simulink model "SdbRxSigProc"  (R2022b) (continued)
== system SdbRxSigProc == (continued)
  HvacFanSts3HvacFanBattI_HvacFanSts3HvacFanBattI/1 -> SdbRxSigProc_Runnable_sys/41(HvacFanSts3HvacFanBattI_HvacFanSts3HvacFanBattI_read)
  HvacFanSts3HvacFanSpdFd_HvacFanSts3HvacFanSpdFd/1 -> SdbRxSigProc_Runnable_sys/42(HvacFanSts3HvacFanSpdFd_HvacFanSts3HvacFanSpdFd_read)
  HvPwrEgyPrio_HvPwrEgyPrio/1 -> SdbRxSigProc_Runnable_sys/43(HvPwrEgyPrio_HvPwrEgyPrio_read)
  IHUAPSts_IHUAPSts/1 -> SdbRxSigProc_Runnable_sys/44(IHUAPSts_IHUAPSts_read)
  IncomingAirQlyCtrlFromHmi_IncomingAirQlyCtrlFromHmi/1 -> SdbRxSigProc_Runnable_sys/45(IncomingAirQlyCtrlFromHmi_IncomingAirQlyCtrlFromHmi_read)
  IncomingAirQlyPopUpReq_IncomingAirQlyPopUpReq/1 -> SdbRxSigProc_Runnable_sys/46(IncomingAirQlyPopUpReq_IncomingAirQlyPopUpReq_read)
  InPm25FanErr_InPm25FanErr/1 -> SdbRxSigProc_Runnable_sys/47(InPm25FanErr_InPm25FanErr_read)
  InPm25SnsrElecErr_InPm25SnsrElecErr/1 -> SdbRxSigProc_Runnable_sys/48(InPm25SnsrElecErr_InPm25SnsrElecErr_read)
  InPm25SnsrSts_InPm25SnsrSts/1 -> SdbRxSigProc_Runnable_sys/49(InPm25SnsrSts_InPm25SnsrSts_read)
  InteCleanUnpleSmell_InteCleanUnpleSmell/1 -> SdbRxSigProc_Runnable_sys/50(InteCleanUnpleSmell_InteCleanUnpleSmell_read)
  IsgMotT_IsgMotT/1 -> SdbRxSigProc_Runnable_sys/51(IsgMotT_IsgMotT_read)
  LeSolarData_LeSolarData/1 -> SdbRxSigProc_Runnable_sys/52(LeSolarData_LeSolarData_read)
  LockgCenSts_LockgCenSts/1 -> SdbRxSigProc_Runnable_sys/53(LockgCenSts_LockgCenSts_read)
  OnBdChrgrHndlSts1_OnBdChrgrHndlSts1/1 -> SdbRxSigProc_Runnable_sys/54(OnBdChrgrHndlSts1_OnBdChrgrHndlSts1_read)
  PassSeatHeatgActlPwrCns_PassSeatHeatgActlPwrCns/1 -> SdbRxSigProc_Runnable_sys/55(PassSeatHeatgActlPwrCns_PassSeatHeatgActlPwrCns_read)
  PassSeatHeatgDesPwrCns_PassSeatHeatgDesPwrCns/1 -> SdbRxSigProc_Runnable_sys/56(PassSeatHeatgDesPwrCns_PassSeatHeatgDesPwrCns_read)
  PassSeatVentnActlPwrCns_PassSeatVentnActlPwrCns/1 -> SdbRxSigProc_Runnable_sys/57(PassSeatVentnActlPwrCns_PassSeatVentnActlPwrCns_read)
  PassSeatVentnDesPwrCns_PassSeatVentnDesPwrCns/1 -> SdbRxSigProc_Runnable_sys/58(PassSeatVentnDesPwrCns_PassSeatVentnDesPwrCns_read)
  PostDrvgClimaReq_PostDrvgClimaReq/1 -> SdbRxSigProc_Runnable_sys/59(PostDrvgClimaReq_PostDrvgClimaReq_read)
  RainfallAmnt_RainfallAmnt/1 -> SdbRxSigProc_Runnable_sys/60(RainfallAmnt_RainfallAmnt_read)
  RelHumSnsrQf_RelHumSnsrQf/1 -> SdbRxSigProc_Runnable_sys/61(RelHumSnsrQf_RelHumSnsrQf_read)
  RemStrtHvCtrlReq_RemStrtHvCtrlReq/1 -> SdbRxSigProc_Runnable_sys/62(RemStrtHvCtrlReq_RemStrtHvCtrlReq_read)
  RiSolarData_RiSolarData/1 -> SdbRxSigProc_Runnable_sys/63(RiSolarData_RiSolarData_read)
  SceneModSeld_SceneModSeld/1 -> SdbRxSigProc_Runnable_sys/64(SceneModSeld_SceneModSeld_read)
  SeatHeatDurgClimaEnadFromTelm_SeatHeatDurgClimaEnadFromTelm/1 -> SdbRxSigProc_Runnable_sys/65(SeatHeatDurgClimaEnadFromTelm_SeatHeatDurgClimaEnadFromTelm_read)
  SecRowSeatHeatgActlPwrCnsLe_SecRowSeatHeatgActlPwrCnsLe/1 -> SdbRxSigProc_Runnable_sys/66(SecRowSeatHeatgActlPwrCnsLe_SecRowSeatHeatgActlPwrCnsLe_read)
  SecRowSeatHeatgActlPwrCnsRi_SecRowSeatHeatgActlPwrCnsRi/1 -> SdbRxSigProc_Runnable_sys/67(SecRowSeatHeatgActlPwrCnsRi_SecRowSeatHeatgActlPwrCnsRi_read)
  SecRowSeatHeatgDesPwrCnsLe_SecRowSeatHeatgDesPwrCnsLe/1 -> SdbRxSigProc_Runnable_sys/68(SecRowSeatHeatgDesPwrCnsLe_SecRowSeatHeatgDesPwrCnsLe_read)
  SecRowSeatHeatgDesPwrCnsRi_SecRowSeatHeatgDesPwrCnsRi/1 -> SdbRxSigProc_Runnable_sys/69(SecRowSeatHeatgDesPwrCnsRi_SecRowSeatHeatgDesPwrCnsRi_read)
  SecRowSeatVentnActlPwrCnsLe_SecRowSeatVentnActlPwrCnsLe/1 -> SdbRxSigProc_Runnable_sys/70(SecRowSeatVentnActlPwrCnsLe_SecRowSeatVentnActlPwrCnsLe_read)
  SecRowSeatVentnActlPwrCnsRi_SecRowSeatVentnActlPwrCnsRi/1 -> SdbRxSigProc_Runnable_sys/71(SecRowSeatVentnActlPwrCnsRi_SecRowSeatVentnActlPwrCnsRi_read)
  SecRowSeatVentnDesPwrCnsLe_SecRowSeatVentnDesPwrCnsLe/1 -> SdbRxSigProc_Runnable_sys/72(SecRowSeatVentnDesPwrCnsLe_SecRowSeatVentnDesPwrCnsLe_read)
  SecRowSeatVentnDesPwrCnsRi_SecRowSeatVentnDesPwrCnsRi/1 -> SdbRxSigProc_Runnable_sys/73(SecRowSeatVentnDesPwrCnsRi_SecRowSeatVentnDesPwrCnsRi_read)
  SolarSnsrVluQf_SolarSnsrVluQf/1 -> SdbRxSigProc_Runnable_sys/74(SolarSnsrVluQf_SolarSnsrVluQf_read)
  SteerWhlHeatgDesPwr_SteerWhlHeatgDesPwr/1 -> SdbRxSigProc_Runnable_sys/75(SteerWhlHeatgDesPwr_SteerWhlHeatgDesPwr_read)
  SteerWhlHeatgPwrAct_SteerWhlHeatgPwrAct/1 -> SdbRxSigProc_Runnable_sys/76(SteerWhlHeatgPwrAct_SteerWhlHeatgPwrAct_read)
  StrtRemSts1WdStrtRemSts_StrtRemSts1WdStrtRemSts/1 -> SdbRxSigProc_Runnable_sys/77(StrtRemSts1WdStrtRemSts_StrtRemSts1WdStrtRemSts_read)
  SunCurtPosnSts_SunCurtPosnSts/1 -> SdbRxSigProc_Runnable_sys/78(SunCurtPosnSts_SunCurtPosnSts_read)
  SunRoofPosnSts_SunRoofPosnSts/1 -> SdbRxSigProc_Runnable_sys/79(SunRoofPosnSts_SunRoofPosnSts_read)
  TelmPM25Req_TelmPM25Req/1 -> SdbRxSigProc_Runnable_sys/80(TelmPM25Req_TelmPM25Req_read)
  TrSts_TrSts/1 -> SdbRxSigProc_Runnable_sys/81(TrSts_TrSts_read)
  VehBattU_VehBattU/1 -> SdbRxSigProc_Runnable_sys/82(VehBattU_VehBattU_read)
  VehSpdLgt_VehSpdLgt/1 -> SdbRxSigProc_Runnable_sys/83(VehSpdLgt_VehSpdLgt_read)
  VehTiAndData_VehTiAndData/1 -> SdbRxSigProc_Runnable_sys/84(VehTiAndData_VehTiAndData_read)
  WadeProtnSts_WadeProtnSts/1 -> SdbRxSigProc_Runnable_sys/85(WadeProtnSts_WadeProtnSts_read)
  WinGlbCmd_WinGlbCmd/1 -> SdbRxSigProc_Runnable_sys/86(WinGlbCmd_WinGlbCmd_read)
  WinPosnStsAtDrvr_WinPosnStsAtDrvr/1 -> SdbRxSigProc_Runnable_sys/87(WinPosnStsAtDrvr_WinPosnStsAtDrvr_read)
  WinPosnStsAtPass_WinPosnStsAtPass/1 -> SdbRxSigProc_Runnable_sys/88(WinPosnStsAtPass_WinPosnStsAtPass_read)
  WinPosnStsAtReLe_WinPosnStsAtReLe/1 -> SdbRxSigProc_Runnable_sys/89(WinPosnStsAtReLe_WinPosnStsAtReLe_read)
  WinPosnStsAtReRi_WinPosnStsAtReRi/1 -> SdbRxSigProc_Runnable_sys/90(WinPosnStsAtReRi_WinPosnStsAtReRi_read)
  WipgInfo_WipgInfo/1 -> SdbRxSigProc_Runnable_sys/91(WipgInfo_WipgInfo_read)
  VehModMngtGlbSafe1_VehModMngtGlbSafe1/1 -> SdbRxSigProc_Runnable_sys/92(VehModMngtGlbSafe1_VehModMngtGlbSafe1_read)
  VehModMngtGlbSafe1_VehModMngtGlbSafe1_IsUpdated/1 -> SdbRxSigProc_Runnable_sys/93(VehModMngtGlbSafe1_VehModMngtGlbSafe1_IsUpdated_read)
  AACAirClngErrSts_AACAirClngErrSts/1 -> SdbRxSigProc_Runnable_sys/94(AACAirClngErrSts_AACAirClngErrSts_read)
  AACAirClngSts_AACAirClngSts/1 -> SdbRxSigProc_Runnable_sys/95(AACAirClngSts_AACAirClngSts_read)
  AFUFragraCh1AvlTiPrec_AFUFragraCh1AvlTiPrec/1 -> SdbRxSigProc_Runnable_sys/96(AFUFragraCh1AvlTiPrec_AFUFragraCh1AvlTiPrec_read)
  AFUFragraCh1AvlTiPrec_AFUFragraCh1AvlTiPrec_IsUpdated/1 -> SdbRxSigProc_Runnable_sys/97(AFUFragraCh1AvlTiPrec_AFUFragraCh1AvlTiPrec_IsUpdated_read)
  AFUFragraCh2AvlTiPrec_AFUFragraCh2AvlTiPrec/1 -> SdbRxSigProc_Runnable_sys/98(AFUFragraCh2AvlTiPrec_AFUFragraCh2AvlTiPrec_read)
  AFUFragraCh2AvlTiPrec_AFUFragraCh2AvlTiPrec_IsUpdated/1 -> SdbRxSigProc_Runnable_sys/99(AFUFragraCh2AvlTiPrec_AFUFragraCh2AvlTiPrec_IsUpdated_read)
  AFUFragraCh3AvlTiPrec_AFUFragraCh3AvlTiPrec/1 -> SdbRxSigProc_Runnable_sys/100(AFUFragraCh3AvlTiPrec_AFUFragraCh3AvlTiPrec_read)
  AFUFragraCh3AvlTiPrec_AFUFragraCh3AvlTiPrec_IsUpdated/1 -> SdbRxSigProc_Runnable_sys/101(AFUFragraCh3AvlTiPrec_AFUFragraCh3AvlTiPrec_IsUpdated_read)
  AFUFragraCh4AvlTiPrec_AFUFragraCh4AvlTiPrec/1 -> SdbRxSigProc_Runnable_sys/102(AFUFragraCh4AvlTiPrec_AFUFragraCh4AvlTiPrec_read)
  AFUFragraCh4AvlTiPrec_AFUFragraCh4AvlTiPrec_IsUpdated/1 -> SdbRxSigProc_Runnable_sys/103(AFUFragraCh4AvlTiPrec_AFUFragraCh4AvlTiPrec_IsUpdated_read)
  AFUFragraCh5AvlTiPrec_AFUFragraCh5AvlTiPrec/1 -> SdbRxSigProc_Runnable_sys/104(AFUFragraCh5AvlTiPrec_AFUFragraCh5AvlTiPrec_read)
  AFUFragraCh5AvlTiPrec_AFUFragraCh5AvlTiPrec_IsUpdated/1 -> SdbRxSigProc_Runnable_sys/105(AFUFragraCh5AvlTiPrec_AFUFragraCh5AvlTiPrec_IsUpdated_read)
  AirFragCh1OvrdDy_AirFragCh1OvrdDy/1 -> SdbRxSigProc_Runnable_sys/106(AirFragCh1OvrdDy_AirFragCh1OvrdDy_read)
  AirFragCh2OvrdDy_AirFragCh2OvrdDy/1 -> SdbRxSigProc_Runnable_sys/107(AirFragCh2OvrdDy_AirFragCh2OvrdDy_read)
  AirFragCh3OvrdDy_AirFragCh3OvrdDy/1 -> SdbRxSigProc_Runnable_sys/108(AirFragCh3OvrdDy_AirFragCh3OvrdDy_read)
  AirFragCh4OvrdDy_AirFragCh4OvrdDy/1 -> SdbRxSigProc_Runnable_sys/109(AirFragCh4OvrdDy_AirFragCh4OvrdDy_read)
  AirFragCh5OvrdDy_AirFragCh5OvrdDy/1 -> SdbRxSigProc_Runnable_sys/110(AirFragCh5OvrdDy_AirFragCh5OvrdDy_read)
  AirPtcEnad_AirPtcEnad/1 -> SdbRxSigProc_Runnable_sys/111(AirPtcEnad_AirPtcEnad_read)
  AirPtcHVPwrActl_AirPtcHVPwrActl/1 -> SdbRxSigProc_Runnable_sys/112(AirPtcHVPwrActl_AirPtcHVPwrActl_read)
  AirPtcHVPwrActlFristLe_AirPtcHVPwrActlFristLe/1 -> SdbRxSigProc_Runnable_sys/113(AirPtcHVPwrActlFristLe_AirPtcHVPwrActlFristLe_read)
  AirPtcHVPwrActlFristRi_AirPtcHVPwrActlFristRi/1 -> SdbRxSigProc_Runnable_sys/114(AirPtcHVPwrActlFristRi_AirPtcHVPwrActlFristRi_read)
  AirPtcHVPwrActlSecLe_AirPtcHVPwrActlSecLe/1 -> SdbRxSigProc_Runnable_sys/115(AirPtcHVPwrActlSecLe_AirPtcHVPwrActlSecLe_read)
  AirPtcModeReq_AirPtcModeReq/1 -> SdbRxSigProc_Runnable_sys/116(AirPtcModeReq_AirPtcModeReq_read)
  AirSrcStsFb_AirSrcStsFb/1 -> SdbRxSigProc_Runnable_sys/117(AirSrcStsFb_AirSrcStsFb_read)
  AltitudeInfrom_AltitudeInfrom/1 -> SdbRxSigProc_Runnable_sys/118(AltitudeInfrom_AltitudeInfrom_read)
  AMBSts_AMBSts/1 -> SdbRxSigProc_Runnable_sys/119(AMBSts_AMBSts_read)
  BackflushVlvErr_BackflushVlvErr/1 -> SdbRxSigProc_Runnable_sys/120(BackflushVlvErr_BackflushVlvErr_read)
  BusCurrentFb_BusCurrentFb/1 -> SdbRxSigProc_Runnable_sys/121(BusCurrentFb_BusCurrentFb_read)
  ChgFilElmWarn_ChgFilElmWarn/1 -> SdbRxSigProc_Runnable_sys/122(ChgFilElmWarn_ChgFilElmWarn_read)
  ClimaCfgForDrvrSeatVentWthSmf2_ClimaCfgForDrvrSeatVentWthSmf2/1 -> SdbRxSigProc_Runnable_sys/123(ClimaCfgForDrvrSeatVentWthSmf2_ClimaCfgForDrvrSeatVentWthSmf2_read)
  ClimaCfgForPassSeatVentWthSmf2_ClimaCfgForPassSeatVentWthSmf2/1 -> SdbRxSigProc_Runnable_sys/124(ClimaCfgForPassSeatVentWthSmf2_ClimaCfgForPassSeatVentWthSmf2_read)
  CmptmtOxygenConcQF_CmptmtOxygenConcQF/1 -> SdbRxSigProc_Runnable_sys/125(CmptmtOxygenConcQF_CmptmtOxygenConcQF_read)
  CmptmtOxygenConcVlu_CmptmtOxygenConcVlu/1 -> SdbRxSigProc_Runnable_sys/126(CmptmtOxygenConcVlu_CmptmtOxygenConcVlu_read)
  CoolgFanErr_CoolgFanErr/1 -> SdbRxSigProc_Runnable_sys/127(CoolgFanErr_CoolgFanErr_read)
  CourseDirFromNav_CourseDirFromNav/1 -> SdbRxSigProc_Runnable_sys/128(CourseDirFromNav_CourseDirFromNav_read)
  CurrentCongestionInformation_CurrentCongestionInformation/1 -> SdbRxSigProc_Runnable_sys/129(CurrentCongestionInformation_CurrentCongestionInformation_read)
  CurrentTunnelInformation_CurrentTunnelInformation/1 -> SdbRxSigProc_Runnable_sys/130(CurrentTunnelInformation_CurrentTunnelInformation_read)
  DcDcActvd_DcDcActvd/1 -> SdbRxSigProc_Runnable_sys/131(DcDcActvd_DcDcActvd_read)
  DcDcPwrLim_DcDcPwrLim/1 -> SdbRxSigProc_Runnable_sys/132(DcDcPwrLim_DcDcPwrLim_read)
  DHUResdSig10_DHUResdSig10/1 -> SdbRxSigProc_Runnable_sys/133(DHUResdSig10_DHUResdSig10_read)
  DHUResdSig11_DHUResdSig11/1 -> SdbRxSigProc_Runnable_sys/134(DHUResdSig11_DHUResdSig11_read)
  DHUResdSig12_DHUResdSig12/1 -> SdbRxSigProc_Runnable_sys/135(DHUResdSig12_DHUResdSig12_read)
  DHUResdSig13_DHUResdSig13/1 -> SdbRxSigProc_Runnable_sys/136(DHUResdSig13_DHUResdSig13_read)
  DHUResdSig3_DHUResdSig3/1 -> SdbRxSigProc_Runnable_sys/137(DHUResdSig3_DHUResdSig3_read)
  DHUResdSig4_DHUResdSig4/1 -> SdbRxSigProc_Runnable_sys/138(DHUResdSig4_DHUResdSig4_read)
  DHUResdSig5_DHUResdSig5/1 -> SdbRxSigProc_Runnable_sys/139(DHUResdSig5_DHUResdSig5_read)
  DHUResdSig6_DHUResdSig6/1 -> SdbRxSigProc_Runnable_sys/140(DHUResdSig6_DHUResdSig6_read)
  DHUResdSig7_DHUResdSig7/1 -> SdbRxSigProc_Runnable_sys/141(DHUResdSig7_DHUResdSig7_read)
  DHUResdSig8_DHUResdSig8/1 -> SdbRxSigProc_Runnable_sys/142(DHUResdSig8_DHUResdSig8_read)
  DHUResdSig9_DHUResdSig9/1 -> SdbRxSigProc_Runnable_sys/143(DHUResdSig9_DHUResdSig9_read)
  DispHvBattLvlOfChrg_DispHvBattLvlOfChrg/1 -> SdbRxSigProc_Runnable_sys/144(DispHvBattLvlOfChrg_DispHvBattLvlOfChrg_read)
  DropTopPosnSts_DropTopPosnSts/1 -> SdbRxSigProc_Runnable_sys/145(DropTopPosnSts_DropTopPosnSts_read)
  DrvModReqType1ForCompile_DrvModReqType1ForCompile/1 -> SdbRxSigProc_Runnable_sys/146(DrvModReqType1ForCompile_DrvModReqType1ForCompile_read)
  DrvrSeatPosPerc_DrvrSeatPosPerc/1 -> SdbRxSigProc_Runnable_sys/147(DrvrSeatPosPerc_DrvrSeatPosPerc_read)
  DrvrSeatPosPerc_DrvrSeatPosPerc_IsUpdated/1 -> SdbRxSigProc_Runnable_sys/148(DrvrSeatPosPerc_DrvrSeatPosPerc_IsUpdated_read)
  DrvrSeatSts_DrvrSeatSts/1 -> SdbRxSigProc_Runnable_sys/149(DrvrSeatSts_DrvrSeatSts_read)
  DrvrSeatVentnActlPwrCns2_DrvrSeatVentnActlPwrCns2/1 -> SdbRxSigProc_Runnable_sys/150(DrvrSeatVentnActlPwrCns2_DrvrSeatVentnActlPwrCns2_read)
  DrvrSeatVentnDesPwrCns2_DrvrSeatVentnDesPwrCns2/1 -> SdbRxSigProc_Runnable_sys/151(DrvrSeatVentnDesPwrCns2_DrvrSeatVentnDesPwrCns2_read)
  DrvrSeatVentnLvlSts2_DrvrSeatVentnLvlSts2/1 -> SdbRxSigProc_Runnable_sys/152(DrvrSeatVentnLvlSts2_DrvrSeatVentnLvlSts2_read)
  EngSt1WdSts_EngSt1WdSts/1 -> SdbRxSigProc_Runnable_sys/153(EngSt1WdSts_EngSt1WdSts_read)
  EngSt1WdSts_EngSt1WdSts_IsUpdated/1 -> SdbRxSigProc_Runnable_sys/154(EngSt1WdSts_EngSt1WdSts_IsUpdated_read)
  FctvSts_FctvSts/1 -> SdbRxSigProc_Runnable_sys/155(FctvSts_FctvSts_read)
  FloatDrvingActv_FloatDrvingActv/1 -> SdbRxSigProc_Runnable_sys/156(FloatDrvingActv_FloatDrvingActv_read)
  FrntAirPtcHVPwrActl_FrntAirPtcHVPwrActl/1 -> SdbRxSigProc_Runnable_sys/157(FrntAirPtcHVPwrActl_FrntAirPtcHVPwrActl_read)
  FrntAirPtcHVPwrActl_FrntAirPtcHVPwrActl_IsUpdated/1 -> SdbRxSigProc_Runnable_sys/158(FrntAirPtcHVPwrActl_FrntAirPtcHVPwrActl_IsUpdated_read)
  HighTempCleanHmiReq_HighTempCleanHmiReq/1 -> SdbRxSigProc_Runnable_sys/159(HighTempCleanHmiReq_HighTempCleanHmiReq_read)
  HmiAC_HmiAC/1 -> SdbRxSigProc_Runnable_sys/160(HmiAC_HmiAC_read)
  HmiAC_HmiAC_IsUpdated/1 -> SdbRxSigProc_Runnable_sys/161(HmiAC_HmiAC_IsUpdated_read)
  HMIAirCdnrAutDrySet_HMIAirCdnrAutDrySet/1 -> SdbRxSigProc_Runnable_sys/162(HMIAirCdnrAutDrySet_HMIAirCdnrAutDrySet_read)
  HMIAirCdnrAutDrySet_HMIAirCdnrAutDrySet_IsUpdated/1 -> SdbRxSigProc_Runnable_sys/163(HMIAirCdnrAutDrySet_HMIAirCdnrAutDrySet_IsUpdated_read)
  HmiAirDistbnRow1_HmiAirDistbnRow1/1 -> SdbRxSigProc_Runnable_sys/164(HmiAirDistbnRow1_HmiAirDistbnRow1_read)
  HmiAirDistbnRow1_HmiAirDistbnRow1_IsUpdated/1 -> SdbRxSigProc_Runnable_sys/165(HmiAirDistbnRow1_HmiAirDistbnRow1_IsUpdated_read)
  HmiAirDistbnRow1Le_HmiAirDistbnRow1Le/1 -> SdbRxSigProc_Runnable_sys/166(HmiAirDistbnRow1Le_HmiAirDistbnRow1Le_read)
  HmiAirDistbnRow1Ri_HmiAirDistbnRow1Ri/1 -> SdbRxSigProc_Runnable_sys/167(HmiAirDistbnRow1Ri_HmiAirDistbnRow1Ri_read)
  HmiAirDistbnRow2_HmiAirDistbnRow2/1 -> SdbRxSigProc_Runnable_sys/168(HmiAirDistbnRow2_HmiAirDistbnRow2_read)
  HmiAirDistbnRow2_HmiAirDistbnRow2_IsUpdated/1 -> SdbRxSigProc_Runnable_sys/169(HmiAirDistbnRow2_HmiAirDistbnRow2_IsUpdated_read)
  HmiAutoRow1_HmiAutoRow1/1 -> SdbRxSigProc_Runnable_sys/170(HmiAutoRow1_HmiAutoRow1_read)
  HmiAutoRow1_HmiAutoRow1_IsUpdated/1 -> SdbRxSigProc_Runnable_sys/171(HmiAutoRow1_HmiAutoRow1_IsUpdated_read)
  HmiAutoRow1Le_HmiAutoRow1Le/1 -> SdbRxSigProc_Runnable_sys/172(HmiAutoRow1Le_HmiAutoRow1Le_read)
  HmiAutoRow1Ri_HmiAutoRow1Ri/1 -> SdbRxSigProc_Runnable_sys/173(HmiAutoRow1Ri_HmiAutoRow1Ri_read)
  HmiAutoRow2_HmiAutoRow2/1 -> SdbRxSigProc_Runnable_sys/174(HmiAutoRow2_HmiAutoRow2_read)
  HmiAutoRow2_HmiAutoRow2_IsUpdated/1 -> SdbRxSigProc_Runnable_sys/175(HmiAutoRow2_HmiAutoRow2_IsUpdated_read)
  HmiAutOxySet_HmiAutOxySet/1 -> SdbRxSigProc_Runnable_sys/176(HmiAutOxySet_HmiAutOxySet_read)
  HMIBluetoothCallToAdjAirVol_HMIBluetoothCallToAdjAirVol/1 -> SdbRxSigProc_Runnable_sys/177(HMIBluetoothCallToAdjAirVol_HMIBluetoothCallToAdjAirVol_read)
  HmiCarLockACRecircSet_HmiCarLockACRecircSet/1 -> SdbRxSigProc_Runnable_sys/178(HmiCarLockACRecircSet_HmiCarLockACRecircSet_read)
  HmiCbnOverHeatPrevnReq_HmiCbnOverHeatPrevnReq/1 -> SdbRxSigProc_Runnable_sys/179(HmiCbnOverHeatPrevnReq_HmiCbnOverHeatPrevnReq_read)
  HmiCbnOverHeatPrevnReq_HmiCbnOverHeatPrevnReq_IsUpdated/1 -> SdbRxSigProc_Runnable_sys/180(HmiCbnOverHeatPrevnReq_HmiCbnOverHeatPrevnReq_IsUpdated_read)
  HmiClimaRow1LePwr_HmiClimaRow1LePwr/1 -> SdbRxSigProc_Runnable_sys/181(HmiClimaRow1LePwr_HmiClimaRow1LePwr_read)
  HmiClimaRow1Pwr_HmiClimaRow1Pwr/1 -> SdbRxSigProc_Runnable_sys/182(HmiClimaRow1Pwr_HmiClimaRow1Pwr_read)
  HmiClimaRow1RiPwr_HmiClimaRow1RiPwr/1 -> SdbRxSigProc_Runnable_sys/183(HmiClimaRow1RiPwr_HmiClimaRow1RiPwr_read)
  HmiClimaRow2Pwr_HmiClimaRow2Pwr/1 -> SdbRxSigProc_Runnable_sys/184(HmiClimaRow2Pwr_HmiClimaRow2Pwr_read)
  HmiClimaRow3Pwr_HmiClimaRow3Pwr/1 -> SdbRxSigProc_Runnable_sys/185(HmiClimaRow3Pwr_HmiClimaRow3Pwr_read)
  HMIClimaVentSet_HMIClimaVentSet/1 -> SdbRxSigProc_Runnable_sys/186(HMIClimaVentSet_HMIClimaVentSet_read)
  HmiCmptmtAirDistbnFrnt_HmiCmptmtAirDistbnFrnt/1 -> SdbRxSigProc_Runnable_sys/187(HmiCmptmtAirDistbnFrnt_HmiCmptmtAirDistbnFrnt_read)
  HmiDrvrHeadPosn_HmiDrvrHeadPosn/1 -> SdbRxSigProc_Runnable_sys/188(HmiDrvrHeadPosn_HmiDrvrHeadPosn_read)
  HmiEcoClimaReq_HmiEcoClimaReq/1 -> SdbRxSigProc_Runnable_sys/189(HmiEcoClimaReq_HmiEcoClimaReq_read)
  HmiEcoClimaReq_HmiEcoClimaReq_IsUpdated/1 -> SdbRxSigProc_Runnable_sys/190(HmiEcoClimaReq_HmiEcoClimaReq_IsUpdated_read)
  HmiElecAirBodyVentLebtn_HmiElecAirBodyVentLebtn/1 -> SdbRxSigProc_Runnable_sys/191(HmiElecAirBodyVentLebtn_HmiElecAirBodyVentLebtn_read)
  HmiElecAirBodyVentRibtn_HmiElecAirBodyVentRibtn/1 -> SdbRxSigProc_Runnable_sys/192(HmiElecAirBodyVentRibtn_HmiElecAirBodyVentRibtn_read)
  HmiElecAirConsolePosn_HmiElecAirConsolePosn/1 -> SdbRxSigProc_Runnable_sys/193(HmiElecAirConsolePosn_HmiElecAirConsolePosn_read)
  HmiElecAirConsoleSwtLe_HmiElecAirConsoleSwtLe/1 -> SdbRxSigProc_Runnable_sys/194(HmiElecAirConsoleSwtLe_HmiElecAirConsoleSwtLe_read)
  HmiElecAirConsoleSwtRi_HmiElecAirConsoleSwtRi/1 -> SdbRxSigProc_Runnable_sys/195(HmiElecAirConsoleSwtRi_HmiElecAirConsoleSwtRi_read)
  HmiElecAirDirModeSecRowReq_HmiElecAirDirModeSecRowReq/1 -> SdbRxSigProc_Runnable_sys/196(HmiElecAirDirModeSecRowReq_HmiElecAirDirModeSecRowReq_read)
  HmiElecAirDirSwngSecLe_HmiElecAirDirSwngSecLe/1 -> SdbRxSigProc_Runnable_sys/197(HmiElecAirDirSwngSecLe_HmiElecAirDirSwngSecLe_read)
  HmiElecAirDirSwngSecRi_HmiElecAirDirSwngSecRi/1 -> SdbRxSigProc_Runnable_sys/198(HmiElecAirDirSwngSecRi_HmiElecAirDirSwngSecRi_read)
  HmiElecAirDrvrNatureWindReq_HmiElecAirDrvrNatureWindReq/1 -> SdbRxSigProc_Runnable_sys/199(HmiElecAirDrvrNatureWindReq_HmiElecAirDrvrNatureWindReq_read)
  HmiElecAirPassNatureWindReq_HmiElecAirPassNatureWindReq/1 -> SdbRxSigProc_Runnable_sys/200(HmiElecAirPassNatureWindReq_HmiElecAirPassNatureWindReq_read)
  HMIFACSwt_HMIFACSwt/1 -> SdbRxSigProc_Runnable_sys/201(HMIFACSwt_HMIFACSwt_read)
  HmiFanLvlRow1_HmiFanLvlRow1/1 -> SdbRxSigProc_Runnable_sys/202(HmiFanLvlRow1_HmiFanLvlRow1_read)
  HmiFanLvlRow1_HmiFanLvlRow1_IsUpdated/1 -> SdbRxSigProc_Runnable_sys/203(HmiFanLvlRow1_HmiFanLvlRow1_IsUpdated_read)
  HmiFanLvlRow1Le_HmiFanLvlRow1Le/1 -> SdbRxSigProc_Runnable_sys/204(HmiFanLvlRow1Le_HmiFanLvlRow1Le_read)
  HmiFanLvlRow1Ri_HmiFanLvlRow1Ri/1 -> SdbRxSigProc_Runnable_sys/205(HmiFanLvlRow1Ri_HmiFanLvlRow1Ri_read)
  HmiFanLvlRow2_HmiFanLvlRow2/1 -> SdbRxSigProc_Runnable_sys/206(HmiFanLvlRow2_HmiFanLvlRow2_read)
  HmiFanLvlRow2_HmiFanLvlRow2_IsUpdated/1 -> SdbRxSigProc_Runnable_sys/207(HmiFanLvlRow2_HmiFanLvlRow2_IsUpdated_read)
  HmiFragraCh_HmiFragraCh/1 -> SdbRxSigProc_Runnable_sys/208(HmiFragraCh_HmiFragraCh_read)
  HmiFragraCh_HmiFragraCh_IsUpdated/1 -> SdbRxSigProc_Runnable_sys/209(HmiFragraCh_HmiFragraCh_IsUpdated_read)
  HmiFragraLvl_HmiFragraLvl/1 -> SdbRxSigProc_Runnable_sys/210(HmiFragraLvl_HmiFragraLvl_read)
  HmiFragraLvl_HmiFragraLvl_IsUpdated/1 -> SdbRxSigProc_Runnable_sys/211(HmiFragraLvl_HmiFragraLvl_IsUpdated_read)
  HMIIonizerAirCdnr_HMIIonizerAirCdnr/1 -> SdbRxSigProc_Runnable_sys/212(HMIIonizerAirCdnr_HMIIonizerAirCdnr_read)
  HMIIonizerAirCdnr_HMIIonizerAirCdnr_IsUpdated/1 -> SdbRxSigProc_Runnable_sys/213(HMIIonizerAirCdnr_HMIIonizerAirCdnr_IsUpdated_read)
  HMILBRACStrtSet_HMILBRACStrtSet/1 -> SdbRxSigProc_Runnable_sys/214(HMILBRACStrtSet_HMILBRACStrtSet_read)
  HmiMaxAC_HmiMaxAC/1 -> SdbRxSigProc_Runnable_sys/215(HmiMaxAC_HmiMaxAC_read)
  HmiMaxAC_HmiMaxAC_IsUpdated/1 -> SdbRxSigProc_Runnable_sys/216(HmiMaxAC_HmiMaxAC_IsUpdated_read)
  HmiMaxDefrst_HmiMaxDefrst/1 -> SdbRxSigProc_Runnable_sys/217(HmiMaxDefrst_HmiMaxDefrst_read)
  HmiMaxDefrst_HmiMaxDefrst_IsUpdated/1 -> SdbRxSigProc_Runnable_sys/218(HmiMaxDefrst_HmiMaxDefrst_IsUpdated_read)
  HmiOxyinCarSwt_HmiOxyinCarSwt/1 -> SdbRxSigProc_Runnable_sys/219(HmiOxyinCarSwt_HmiOxyinCarSwt_read)
  HmiParkVentSet_HmiParkVentSet/1 -> SdbRxSigProc_Runnable_sys/220(HmiParkVentSet_HmiParkVentSet_read)
  HmiParkVentTmrSet_HmiParkVentTmrSet/1 -> SdbRxSigProc_Runnable_sys/221(HmiParkVentTmrSet_HmiParkVentTmrSet_read)
  HmiPassHeadPosn_HmiPassHeadPosn/1 -> SdbRxSigProc_Runnable_sys/222(HmiPassHeadPosn_HmiPassHeadPosn_read)
  HmiPM25PopUpResp_HmiPM25PopUpResp/1 -> SdbRxSigProc_Runnable_sys/223(HmiPM25PopUpResp_HmiPM25PopUpResp_read)
  HmiPM25PopUpResp_HmiPM25PopUpResp_IsUpdated/1 -> SdbRxSigProc_Runnable_sys/224(HmiPM25PopUpResp_HmiPM25PopUpResp_IsUpdated_read)
  HmiPM25PopUpSet_HmiPM25PopUpSet/1 -> SdbRxSigProc_Runnable_sys/225(HmiPM25PopUpSet_HmiPM25PopUpSet_read)
  HmiPM25PopUpSet_HmiPM25PopUpSet_IsUpdated/1 -> SdbRxSigProc_Runnable_sys/226(HmiPM25PopUpSet_HmiPM25PopUpSet_IsUpdated_read)
  HmiSmrtWindFolwSet_HmiSmrtWindFolwSet/1 -> SdbRxSigProc_Runnable_sys/227(HmiSmrtWindFolwSet_HmiSmrtWindFolwSet_read)
  HMIThermoTankDoorOpen_HMIThermoTankDoorOpen/1 -> SdbRxSigProc_Runnable_sys/228(HMIThermoTankDoorOpen_HMIThermoTankDoorOpen_read)
  HMIThermoTankEcoSw_HMIThermoTankEcoSw/1 -> SdbRxSigProc_Runnable_sys/229(HMIThermoTankEcoSw_HMIThermoTankEcoSw_read)
  HMIThermoTankT_HMIThermoTankT/1 -> SdbRxSigProc_Runnable_sys/230(HMIThermoTankT_HMIThermoTankT_read)
  HmiThermoTSteDeoNewSwt_HmiThermoTSteDeoNewSwt/1 -> SdbRxSigProc_Runnable_sys/231(HmiThermoTSteDeoNewSwt_HmiThermoTSteDeoNewSwt_read)
  HmiThermoTSteDeoSwt_HmiThermoTSteDeoSwt/1 -> SdbRxSigProc_Runnable_sys/232(HmiThermoTSteDeoSwt_HmiThermoTSteDeoSwt_read)
  HmiTSyncn_HmiTSyncn/1 -> SdbRxSigProc_Runnable_sys/233(HmiTSyncn_HmiTSyncn_read)
  HmiTSyncn_HmiTSyncn_IsUpdated/1 -> SdbRxSigProc_Runnable_sys/234(HmiTSyncn_HmiTSyncn_IsUpdated_read)
  HmiUVSterilSet_HmiUVSterilSet/1 -> SdbRxSigProc_Runnable_sys/235(HmiUVSterilSet_HmiUVSterilSet_read)
  HmiWinterLockCarPrevnIceSet_HmiWinterLockCarPrevnIceSet/1 -> SdbRxSigProc_Runnable_sys/236(HmiWinterLockCarPrevnIceSet_HmiWinterLockCarPrevnIceSet_read)
  HmiWinterLockCarPrevnIceSet_HmiWinterLockCarPrevnIceSet_IsUpdated/1 -> SdbRxSigProc_Runnable_sys/237(HmiWinterLockCarPrevnIceSet_HmiWinterLockCarPrevnIceSet_IsUpdated_read)
  HoodSts_HoodSts/1 -> SdbRxSigProc_Runnable_sys/238(HoodSts_HoodSts_read)
  HvBattLimnIndcn_HvBattLimnIndcn/1 -> SdbRxSigProc_Runnable_sys/239(HvBattLimnIndcn_HvBattLimnIndcn_read)
  InflateAirSrcSwt_InflateAirSrcSwt/1 -> SdbRxSigProc_Runnable_sys/240(InflateAirSrcSwt_InflateAirSrcSwt_read)
  InpVoltFb_InpVoltFb/1 -> SdbRxSigProc_Runnable_sys/241(InpVoltFb_InpVoltFb_read)
  LiOprnMod_LiOprnMod/1 -> SdbRxSigProc_Runnable_sys/242(LiOprnMod_LiOprnMod_read)
  LonglifeBatteryReq_LonglifeBatteryReq/1 -> SdbRxSigProc_Runnable_sys/243(LonglifeBatteryReq_LonglifeBatteryReq_read)
  McuOvrTempErr_McuOvrTempErr/1 -> SdbRxSigProc_Runnable_sys/244(McuOvrTempErr_McuOvrTempErr_read)
  McuTempFb_McuTempFb/1 -> SdbRxSigProc_Runnable_sys/245(McuTempFb_McuTempFb_read)
  McuTempSnsrErr_McuTempSnsrErr/1 -> SdbRxSigProc_Runnable_sys/246(McuTempSnsrErr_McuTempSnsrErr_read)
  MoleculeVlv1_MoleculeVlv1/1 -> SdbRxSigProc_Runnable_sys/247(MoleculeVlv1_MoleculeVlv1_read)
  MoleculeVlv2_MoleculeVlv2/1 -> SdbRxSigProc_Runnable_sys/248(MoleculeVlv2_MoleculeVlv2_read)
  MosOvrTempErr_MosOvrTempErr/1 -> SdbRxSigProc_Runnable_sys/249(MosOvrTempErr_MosOvrTempErr_read)
  MosTempFb_MosTempFb/1 -> SdbRxSigProc_Runnable_sys/250(MosTempFb_MosTempFb_read)
  MosTempSnsrErr_MosTempSnsrErr/1 -> SdbRxSigProc_Runnable_sys/251(MosTempSnsrErr_MosTempSnsrErr_read)
  MotStsFrmOGM_MotStsFrmOGM/1 -> SdbRxSigProc_Runnable_sys/252(MotStsFrmOGM_MotStsFrmOGM_read)
  MotTempFb_MotTempFb/1 -> SdbRxSigProc_Runnable_sys/253(MotTempFb_MotTempFb_read)
  MotTempSnsrErr_MotTempSnsrErr/1 -> SdbRxSigProc_Runnable_sys/254(MotTempSnsrErr_MotTempSnsrErr_read)
  OGMCmprSpdFb_OGMCmprSpdFb/1 -> SdbRxSigProc_Runnable_sys/255(OGMCmprSpdFb_OGMCmprSpdFb_read)
  OGMFlowFb_OGMFlowFb/1 -> SdbRxSigProc_Runnable_sys/256(OGMFlowFb_OGMFlowFb_read)
  OGMHwVers_OGMHwVers/1 -> SdbRxSigProc_Runnable_sys/257(OGMHwVers_OGMHwVers_read)
  OGMIntErr_OGMIntErr/1 -> SdbRxSigProc_Runnable_sys/258(OGMIntErr_OGMIntErr_read)
  OGMOvrVoltRangErr_OGMOvrVoltRangErr/1 -> SdbRxSigProc_Runnable_sys/259(OGMOvrVoltRangErr_OGMOvrVoltRangErr_read)
  OGMRunTi_OGMRunTi/1 -> SdbRxSigProc_Runnable_sys/260(OGMRunTi_OGMRunTi_read)
  OGMSwVers_OGMSwVers/1 -> SdbRxSigProc_Runnable_sys/261(OGMSwVers_OGMSwVers_read)
  OGMTempErr_OGMTempErr/1 -> SdbRxSigProc_Runnable_sys/262(OGMTempErr_OGMTempErr_read)
  OGMUndVoltRangErr_OGMUndVoltRangErr/1 -> SdbRxSigProc_Runnable_sys/263(OGMUndVoltRangErr_OGMUndVoltRangErr_read)
  OxygenConcFbFrmOGM_OxygenConcFbFrmOGM/1 -> SdbRxSigProc_Runnable_sys/264(OxygenConcFbFrmOGM_OxygenConcFbFrmOGM_read)
  OxygenConcFlt_OxygenConcFlt/1 -> SdbRxSigProc_Runnable_sys/265(OxygenConcFlt_OxygenConcFlt_read)
  OxygenConcSnsrElecErr_OxygenConcSnsrElecErr/1 -> SdbRxSigProc_Runnable_sys/266(OxygenConcSnsrElecErr_OxygenConcSnsrElecErr_read)
  OxygenConcSnsrIntErr_OxygenConcSnsrIntErr/1 -> SdbRxSigProc_Runnable_sys/267(OxygenConcSnsrIntErr_OxygenConcSnsrIntErr_read)
  OxygenConcSnsrOvrVoltRangErr_OxygenConcSnsrOvrVoltRangErr/1 -> SdbRxSigProc_Runnable_sys/268(OxygenConcSnsrOvrVoltRangErr_OxygenConcSnsrOvrVoltRangErr_read)
  OxygenConcSnsrSts_OxygenConcSnsrSts/1 -> SdbRxSigProc_Runnable_sys/269(OxygenConcSnsrSts_OxygenConcSnsrSts_read)
  OxygenConcSnsrStsFrmOGM_OxygenConcSnsrStsFrmOGM/1 -> SdbRxSigProc_Runnable_sys/270(OxygenConcSnsrStsFrmOGM_OxygenConcSnsrStsFrmOGM_read)
  OxygenConcSnsrTempErr_OxygenConcSnsrTempErr/1 -> SdbRxSigProc_Runnable_sys/271(OxygenConcSnsrTempErr_OxygenConcSnsrTempErr_read)
  OxygenConcSnsrUndVoltRangErr_OxygenConcSnsrUndVoltRangErr/1 -> SdbRxSigProc_Runnable_sys/272(OxygenConcSnsrUndVoltRangErr_OxygenConcSnsrUndVoltRangErr_read)
  OxygenGenrErrInfo_OxygenGenrErrInfo/1 -> SdbRxSigProc_Runnable_sys/273(OxygenGenrErrInfo_OxygenGenrErrInfo_read)
  OxygenSplyAvlSts_OxygenSplyAvlSts/1 -> SdbRxSigProc_Runnable_sys/274(OxygenSplyAvlSts_OxygenSplyAvlSts_read)
  OxygenSplyErr_OxygenSplyErr/1 -> SdbRxSigProc_Runnable_sys/275(OxygenSplyErr_OxygenSplyErr_read)
  OxygenTubeBlk_OxygenTubeBlk/1 -> SdbRxSigProc_Runnable_sys/276(OxygenTubeBlk_OxygenTubeBlk_read)
  PassSeatPosPerc_PassSeatPosPerc/1 -> SdbRxSigProc_Runnable_sys/277(PassSeatPosPerc_PassSeatPosPerc_read)
  PassSeatPosPerc_PassSeatPosPerc_IsUpdated/1 -> SdbRxSigProc_Runnable_sys/278(PassSeatPosPerc_PassSeatPosPerc_IsUpdated_read)
  PassSeatSts2_PassSeatSts2/1 -> SdbRxSigProc_Runnable_sys/279(PassSeatSts2_PassSeatSts2_read)
  PassSeatVentnActlPwrCns2_PassSeatVentnActlPwrCns2/1 -> SdbRxSigProc_Runnable_sys/280(PassSeatVentnActlPwrCns2_PassSeatVentnActlPwrCns2_read)
  PassSeatVentnDesPwrCns2_PassSeatVentnDesPwrCns2/1 -> SdbRxSigProc_Runnable_sys/281(PassSeatVentnDesPwrCns2_PassSeatVentnDesPwrCns2_read)
  PassSeatVentnLvlSts2_PassSeatVentnLvlSts2/1 -> SdbRxSigProc_Runnable_sys/282(PassSeatVentnLvlSts2_PassSeatVentnLvlSts2_read)
  PhaseCurrentFb_PhaseCurrentFb/1 -> SdbRxSigProc_Runnable_sys/283(PhaseCurrentFb_PhaseCurrentFb_read)
  PosnBrief_PosnBrief/1 -> SdbRxSigProc_Runnable_sys/284(PosnBrief_PosnBrief_read)
  PressureFb_PressureFb/1 -> SdbRxSigProc_Runnable_sys/285(PressureFb_PressureFb_read)
  PressureFlt_PressureFlt/1 -> SdbRxSigProc_Runnable_sys/286(PressureFlt_PressureFlt_read)
  PressureSnsrErr_PressureSnsrErr/1 -> SdbRxSigProc_Runnable_sys/287(PressureSnsrErr_PressureSnsrErr_read)
  RefrLpSwSts_RefrLpSwSts/1 -> SdbRxSigProc_Runnable_sys/288(RefrLpSwSts_RefrLpSwSts_read)
  RefrtorVlvEna_RefrtorVlvEna/1 -> SdbRxSigProc_Runnable_sys/289(RefrtorVlvEna_RefrtorVlvEna_read)
  ResrvdSigForCCM2_ResrvdSigForCCM2/1 -> SdbRxSigProc_Runnable_sys/290(ResrvdSigForCCM2_ResrvdSigForCCM2_read)
  RoadIncln_RoadIncln/1 -> SdbRxSigProc_Runnable_sys/291(RoadIncln_RoadIncln_read)
  RuranACModeSet_RuranACModeSet/1 -> SdbRxSigProc_Runnable_sys/292(RuranACModeSet_RuranACModeSet_read)
  SeatBackAngleRowFirstDrvr_SeatBackAngleRowFirstDrvr/1 -> SdbRxSigProc_Runnable_sys/293(SeatBackAngleRowFirstDrvr_SeatBackAngleRowFirstDrvr_read)
  SeatBackAngleRowFirstDrvr_SeatBackAngleRowFirstDrvr_IsUpdated/1 -> SdbRxSigProc_Runnable_sys/294(SeatBackAngleRowFirstDrvr_SeatBackAngleRowFirstDrvr_IsUpdated_read)
  SeatBackAngleRowFirstPass_SeatBackAngleRowFirstPass/1 -> SdbRxSigProc_Runnable_sys/295(SeatBackAngleRowFirstPass_SeatBackAngleRowFirstPass_read)
  SeatBackAngleRowFirstPass_SeatBackAngleRowFirstPass_IsUpdated/1 -> SdbRxSigProc_Runnable_sys/296(SeatBackAngleRowFirstPass_SeatBackAngleRowFirstPass_IsUpdated_read)
  SeatOccptAtRowSecDrSide2_SeatOccptAtRowSecDrSide2/1 -> SdbRxSigProc_Runnable_sys/297(SeatOccptAtRowSecDrSide2_SeatOccptAtRowSecDrSide2_read)
  SeatOccptAtRowSecMid2_SeatOccptAtRowSecMid2/1 -> SdbRxSigProc_Runnable_sys/298(SeatOccptAtRowSecMid2_SeatOccptAtRowSecMid2_read)
  SeatOccptAtRowSecPasSide2_SeatOccptAtRowSecPasSide2/1 -> SdbRxSigProc_Runnable_sys/299(SeatOccptAtRowSecPasSide2_SeatOccptAtRowSecPasSide2_read)
  SeatOccptAtRowThrdLe2_SeatOccptAtRowThrdLe2/1 -> SdbRxSigProc_Runnable_sys/300(SeatOccptAtRowThrdLe2_SeatOccptAtRowThrdLe2_read)
  SeatOccptAtRowThrdRi2_SeatOccptAtRowThrdRi2/1 -> SdbRxSigProc_Runnable_sys/301(SeatOccptAtRowThrdRi2_SeatOccptAtRowThrdRi2_read)
  SportModeThmgtRequest_SportModeThmgtRequest/1 -> SdbRxSigProc_Runnable_sys/302(SportModeThmgtRequest_SportModeThmgtRequest_read)
  TCSCooltFlow_TCSCooltFlow/1 -> SdbRxSigProc_Runnable_sys/303(TCSCooltFlow_TCSCooltFlow_read)
  TCSCooltT_TCSCooltT/1 -> SdbRxSigProc_Runnable_sys/304(TCSCooltT_TCSCooltT_read)
  TcsDoorEleBailReq_TcsDoorEleBailReq/1 -> SdbRxSigProc_Runnable_sys/305(TcsDoorEleBailReq_TcsDoorEleBailReq_read)
  TcsFanReq_TcsFanReq/1 -> SdbRxSigProc_Runnable_sys/306(TcsFanReq_TcsFanReq_read)
  TcsModSwtReq_TcsModSwtReq/1 -> SdbRxSigProc_Runnable_sys/307(TcsModSwtReq_TcsModSwtReq_read)
  TcsOvrheatdProtnSts_TcsOvrheatdProtnSts/1 -> SdbRxSigProc_Runnable_sys/308(TcsOvrheatdProtnSts_TcsOvrheatdProtnSts_read)
  TcsSemiconReq_TcsSemiconReq/1 -> SdbRxSigProc_Runnable_sys/309(TcsSemiconReq_TcsSemiconReq_read)
  TcsTmrOverReq_TcsTmrOverReq/1 -> SdbRxSigProc_Runnable_sys/310(TcsTmrOverReq_TcsTmrOverReq_read)
  TcsTNowCtrlReq_TcsTNowCtrlReq/1 -> SdbRxSigProc_Runnable_sys/311(TcsTNowCtrlReq_TcsTNowCtrlReq_read)
  TcsTopDoorEleBailReq_TcsTopDoorEleBailReq/1 -> SdbRxSigProc_Runnable_sys/312(TcsTopDoorEleBailReq_TcsTopDoorEleBailReq_read)
  TelmOxygenValReq_TelmOxygenValReq/1 -> SdbRxSigProc_Runnable_sys/313(TelmOxygenValReq_TelmOxygenValReq_read)
  TelmThermoTankECOSet_TelmThermoTankECOSet/1 -> SdbRxSigProc_Runnable_sys/314(TelmThermoTankECOSet_TelmThermoTankECOSet_read)
  TelmThermoTankT_TelmThermoTankT/1 -> SdbRxSigProc_Runnable_sys/315(TelmThermoTankT_TelmThermoTankT_read)
  ThermHvacRecircReq_ThermHvacRecircReq/1 -> SdbRxSigProc_Runnable_sys/316(ThermHvacRecircReq_ThermHvacRecircReq_read)
  ThermHvacRecircReqEna_ThermHvacRecircReqEna/1 -> SdbRxSigProc_Runnable_sys/317(ThermHvacRecircReqEna_ThermHvacRecircReqEna_read)
  ThrdRowSeatHeatgActlPwrCnsLe_ThrdRowSeatHeatgActlPwrCnsLe/1 -> SdbRxSigProc_Runnable_sys/318(ThrdRowSeatHeatgActlPwrCnsLe_ThrdRowSeatHeatgActlPwrCnsLe_read)
  ThrdRowSeatHeatgActlPwrCnsRi_ThrdRowSeatHeatgActlPwrCnsRi/1 -> SdbRxSigProc_Runnable_sys/319(ThrdRowSeatHeatgActlPwrCnsRi_ThrdRowSeatHeatgActlPwrCnsRi_read)
  ThrdRowSeatHeatgDesPwrCnsLe_ThrdRowSeatHeatgDesPwrCnsLe/1 -> SdbRxSigProc_Runnable_sys/320(ThrdRowSeatHeatgDesPwrCnsLe_ThrdRowSeatHeatgDesPwrCnsLe_read)
  ThrdRowSeatHeatgDesPwrCnsRi_ThrdRowSeatHeatgDesPwrCnsRi/1 -> SdbRxSigProc_Runnable_sys/321(ThrdRowSeatHeatgDesPwrCnsRi_ThrdRowSeatHeatgDesPwrCnsRi_read)
  ThrdRowSeatVentnActlPwrCnsLe_ThrdRowSeatVentnActlPwrCnsLe/1 -> SdbRxSigProc_Runnable_sys/322(ThrdRowSeatVentnActlPwrCnsLe_ThrdRowSeatVentnActlPwrCnsLe_read)
  ThrdRowSeatVentnActlPwrCnsRi_ThrdRowSeatVentnActlPwrCnsRi/1 -> SdbRxSigProc_Runnable_sys/323(ThrdRowSeatVentnActlPwrCnsRi_ThrdRowSeatVentnActlPwrCnsRi_read)
  ThrdRowSeatVentnDesPwrCnsLe_ThrdRowSeatVentnDesPwrCnsLe/1 -> SdbRxSigProc_Runnable_sys/324(ThrdRowSeatVentnDesPwrCnsLe_ThrdRowSeatVentnDesPwrCnsLe_read)
  ThrdRowSeatVentnDesPwrCnsRi_ThrdRowSeatVentnDesPwrCnsRi/1 -> SdbRxSigProc_Runnable_sys/325(ThrdRowSeatVentnDesPwrCnsRi_ThrdRowSeatVentnDesPwrCnsRi_read)
  UseEvapReqTMinNew_UseEvapReqTMinNew/1 -> SdbRxSigProc_Runnable_sys/326(UseEvapReqTMinNew_UseEvapReqTMinNew_read)
  VlvVoltFlt_VlvVoltFlt/1 -> SdbRxSigProc_Runnable_sys/327(VlvVoltFlt_VlvVoltFlt_read)
  WarnLvlMsg_WarnLvlMsg/1 -> SdbRxSigProc_Runnable_sys/328(WarnLvlMsg_WarnLvlMsg_read)
  WarnLvlMsg_WarnLvlMsg_IsUpdated/1 -> SdbRxSigProc_Runnable_sys/329(WarnLvlMsg_WarnLvlMsg_IsUpdated_read)
  WasRespLevel_WasRespLevel/1 -> SdbRxSigProc_Runnable_sys/330(WasRespLevel_WasRespLevel_read)
  WasRespLevel_WasRespLevel_IsUpdated/1 -> SdbRxSigProc_Runnable_sys/331(WasRespLevel_WasRespLevel_IsUpdated_read)
  WaterDepthInformation_WaterDepthInformation/1 -> SdbRxSigProc_Runnable_sys/332(WaterDepthInformation_WaterDepthInformation_read)
  WeatherQualityAQI_WeatherQualityAQI/1 -> SdbRxSigProc_Runnable_sys/333(WeatherQualityAQI_WeatherQualityAQI_read)
  SdbRxSigProc_Runnable_sys/1(AACAirClngErrStsNgcc_AACAirClngErrStsNgcc_write) -> AACAirClngErrStsNgcc_AACAirClngErrStsNgcc/1
  SdbRxSigProc_Runnable_sys/2(AACAirClngStsNgcc_AACAirClngStsNgcc_write) -> AACAirClngStsNgcc_AACAirClngStsNgcc/1
  SdbRxSigProc_Runnable_sys/3(AFUFragraCh1AvlTiPrecNgcc_AFUFragraCh1AvlTiPrecNgcc_write) -> AFUFragraCh1AvlTiPrecNgcc_AFUFragraCh1AvlTiPrecNgcc/1
  SdbRxSigProc_Runnable_sys/4(AFUFragraCh2AvlTiPrecNgcc_AFUFragraCh2AvlTiPrecNgcc_write) -> AFUFragraCh2AvlTiPrecNgcc_AFUFragraCh2AvlTiPrecNgcc/1
  SdbRxSigProc_Runnable_sys/5(AFUFragraCh3AvlTiPrecNgcc_AFUFragraCh3AvlTiPrecNgcc_write) -> AFUFragraCh3AvlTiPrecNgcc_AFUFragraCh3AvlTiPrecNgcc/1
  SdbRxSigProc_Runnable_sys/6(AFUFragraCh4AvlTiPrecNgcc_AFUFragraCh4AvlTiPrecNgcc_write) -> AFUFragraCh4AvlTiPrecNgcc_AFUFragraCh4AvlTiPrecNgcc/1
  SdbRxSigProc_Runnable_sys/7(AFUFragraCh5AvlTiPrecNgcc_AFUFragraCh5AvlTiPrecNgcc_write) -> AFUFragraCh5AvlTiPrecNgcc_AFUFragraCh5AvlTiPrecNgcc/1
  SdbRxSigProc_Runnable_sys/8(AirClngReq_AirClngReq_write) -> AirClngReq_AirClngReq/1
  SdbRxSigProc_Runnable_sys/9(AirFragCh1OvrdDyNgcc_AirFragCh1OvrdDyNgcc_write) -> AirFragCh1OvrdDyNgcc_AirFragCh1OvrdDyNgcc/1
  SdbRxSigProc_Runnable_sys/10(AirFragCh2OvrdDyNgcc_AirFragCh2OvrdDyNgcc_write) -> AirFragCh2OvrdDyNgcc_AirFragCh2OvrdDyNgcc/1
  SdbRxSigProc_Runnable_sys/11(AirFragCh3OvrdDyNgcc_AirFragCh3OvrdDyNgcc_write) -> AirFragCh3OvrdDyNgcc_AirFragCh3OvrdDyNgcc/1
  SdbRxSigProc_Runnable_sys/12(AirFragCh4OvrdDyNgcc_AirFragCh4OvrdDyNgcc_write) -> AirFragCh4OvrdDyNgcc_AirFragCh4OvrdDyNgcc/1
  SdbRxSigProc_Runnable_sys/13(AirFragCh5OvrdDyNgcc_AirFragCh5OvrdDyNgcc_write) -> AirFragCh5OvrdDyNgcc_AirFragCh5OvrdDyNgcc/1
  SdbRxSigProc_Runnable_sys/14(AirPtcEnadNgcc_AirPtcEnadNgcc_write) -> AirPtcEnadNgcc_AirPtcEnadNgcc/1
  SdbRxSigProc_Runnable_sys/15(AirPtcHVPwrActlFirstLeNgcc_AirPtcHVPwrActlFirstLeNgcc_write) -> AirPtcHVPwrActlFirstLeNgcc_AirPtcHVPwrActlFirstLeNgcc/1
  SdbRxSigProc_Runnable_sys/16(AirPtcHVPwrActlFirstRiNgcc_AirPtcHVPwrActlFirstRiNgcc_write) -> AirPtcHVPwrActlFirstRiNgcc_AirPtcHVPwrActlFirstRiNgcc/1
  SdbRxSigProc_Runnable_sys/17(AirPtcHVPwrActlSecLeNgcc_AirPtcHVPwrActlSecLeNgcc_write) -> AirPtcHVPwrActlSecLeNgcc_AirPtcHVPwrActlSecLeNgcc/1
  SdbRxSigProc_Runnable_sys/18(AirPtcModeReqNgcc_AirPtcModeReqNgcc_write) -> AirPtcModeReqNgcc_AirPtcModeReqNgcc/1
  SdbRxSigProc_Runnable_sys/19(AirSrcStsFbNgcc_AirSrcStsFbNgcc_write) -> AirSrcStsFbNgcc_AirSrcStsFbNgcc/1
  SdbRxSigProc_Runnable_sys/20(AltitudeInfromNgcc_AltitudeInfromNgcc_write) -> AltitudeInfromNgcc_AltitudeInfromNgcc/1
  SdbRxSigProc_Runnable_sys/21(AMBStsNgcc_AMBStsNgcc_write) -> AMBStsNgcc_AMBStsNgcc/1
  SdbRxSigProc_Runnable_sys/22(BackflushVlvErrNgcc_BackflushVlvErrNgcc_write) -> BackflushVlvErrNgcc_BackflushVlvErrNgcc/1
  SdbRxSigProc_Runnable_sys/23(BookTrvlClimaActvReq_Ngcc_BookTrvlClimaActvReq_write) -> BookTrvlClimaActvReq_Ngcc_BookTrvlClimaActvReq/1
  SdbRxSigProc_Runnable_sys/24(BusCurrentFbNgcc_BusCurrentFbNgcc_write) -> BusCurrentFbNgcc_BusCurrentFbNgcc/1
  SdbRxSigProc_Runnable_sys/25(ChgFilElmWarnNgcc_ChgFilElmWarnNgcc_write) -> ChgFilElmWarnNgcc_ChgFilElmWarnNgcc/1
  SdbRxSigProc_Runnable_sys/26(CmptmtOxygenConcQFNgcc_CmptmtOxygenConcQFNgcc_write) -> CmptmtOxygenConcQFNgcc_CmptmtOxygenConcQFNgcc/1
  SdbRxSigProc_Runnable_sys/27(CmptmtOxygenConcVluNgcc_CmptmtOxygenConcVluNgcc_write) -> CmptmtOxygenConcVluNgcc_CmptmtOxygenConcVluNgcc/1
  SdbRxSigProc_Runnable_sys/28(CoolgFanErrNgcc_CoolgFanErrNgcc_write) -> CoolgFanErrNgcc_CoolgFanErrNgcc/1
  SdbRxSigProc_Runnable_sys/29(CourseDirFromNavNgcc_CourseDirFromNavNgcc_write) -> CourseDirFromNavNgcc_CourseDirFromNavNgcc/1
  SdbRxSigProc_Runnable_sys/30(CurrentCongestionInformationNgcc_CurrentCongestionInformationNgcc_write) -> CurrentCongestionInformationNgcc_CurrentCongestionInformationNgcc/1
  SdbRxSigProc_Runnable_sys/31(CurrentTunnelInformationNgcc_CurrentTunnelInformationNgcc_write) -> CurrentTunnelInformationNgcc_CurrentTunnelInformationNgcc/1
  SdbRxSigProc_Runnable_sys/32(DcDcActvdNgcc_DcDcActvdNgcc_write) -> DcDcActvdNgcc_DcDcActvdNgcc/1
  SdbRxSigProc_Runnable_sys/33(DcDcPwrLimNgcc_DcDcPwrLimNgcc_write) -> DcDcPwrLimNgcc_DcDcPwrLimNgcc/1
  SdbRxSigProc_Runnable_sys/34(DHUResdSig10Ngcc_DHUResdSig10Ngcc_write) -> DHUResdSig10Ngcc_DHUResdSig10Ngcc/1
  SdbRxSigProc_Runnable_sys/35(DHUResdSig11Ngcc_DHUResdSig11Ngcc_write) -> DHUResdSig11Ngcc_DHUResdSig11Ngcc/1
  SdbRxSigProc_Runnable_sys/36(DHUResdSig12Ngcc_DHUResdSig12Ngcc_write) -> DHUResdSig12Ngcc_DHUResdSig12Ngcc/1
  SdbRxSigProc_Runnable_sys/37(DHUResdSig13Ngcc_DHUResdSig13Ngcc_write) -> DHUResdSig13Ngcc_DHUResdSig13Ngcc/1
  SdbRxSigProc_Runnable_sys/38(DHUResdSig3Ngcc_DHUResdSig3Ngcc_write) -> DHUResdSig3Ngcc_DHUResdSig3Ngcc/1
  SdbRxSigProc_Runnable_sys/39(DHUResdSig4Ngcc_DHUResdSig4Ngcc_write) -> DHUResdSig4Ngcc_DHUResdSig4Ngcc/1
  SdbRxSigProc_Runnable_sys/40(DHUResdSig5Ngcc_DHUResdSig5Ngcc_write) -> DHUResdSig5Ngcc_DHUResdSig5Ngcc/1
  SdbRxSigProc_Runnable_sys/41(DHUResdSig6Ngcc_DHUResdSig6Ngcc_write) -> DHUResdSig6Ngcc_DHUResdSig6Ngcc/1
  SdbRxSigProc_Runnable_sys/42(DHUResdSig7Ngcc_DHUResdSig7Ngcc_write) -> DHUResdSig7Ngcc_DHUResdSig7Ngcc/1
  SdbRxSigProc_Runnable_sys/43(DHUResdSig8Ngcc_DHUResdSig8Ngcc_write) -> DHUResdSig8Ngcc_DHUResdSig8Ngcc/1
  SdbRxSigProc_Runnable_sys/44(DHUResdSig9Ngcc_DHUResdSig9Ngcc_write) -> DHUResdSig9Ngcc_DHUResdSig9Ngcc/1
  SdbRxSigProc_Runnable_sys/45(DispHvBattLvlOfChrgNgcc_DispHvBattLvlOfChrgNgcc_write) -> DispHvBattLvlOfChrgNgcc_DispHvBattLvlOfChrgNgcc/1
  SdbRxSigProc_Runnable_sys/46(DropTopPosnStsNgcc_DropTopPosnStsNgcc_write) -> DropTopPosnStsNgcc_DropTopPosnStsNgcc/1
  SdbRxSigProc_Runnable_sys/47(DrvrSeatPosPercNgcc_DrvrSeatPosPercNgcc_write) -> DrvrSeatPosPercNgcc_DrvrSeatPosPercNgcc/1
  SdbRxSigProc_Runnable_sys/48(DrvrSeatSts_Ngcc_DrvrSeatSts_write) -> DrvrSeatSts_Ngcc_DrvrSeatSts/1
  SdbRxSigProc_Runnable_sys/49(DrvrSeatVentnLvlSts2Ngcc_DrvrSeatVentnLvlSts2Ngcc_write) -> DrvrSeatVentnLvlSts2Ngcc_DrvrSeatVentnLvlSts2Ngcc/1
  SdbRxSigProc_Runnable_sys/50(FctvStsNgcc_FctvStsNgcc_write) -> FctvStsNgcc_FctvStsNgcc/1
  SdbRxSigProc_Runnable_sys/51(FloatDrvingActvNgcc_FloatDrvingActvNgcc_write) -> FloatDrvingActvNgcc_FloatDrvingActvNgcc/1
  SdbRxSigProc_Runnable_sys/52(HighTempCleanHmiReqNgcc_HighTempCleanHmiReqNgcc_write) -> HighTempCleanHmiReqNgcc_HighTempCleanHmiReqNgcc/1
  SdbRxSigProc_Runnable_sys/53(HMIAirCdnrAutDrySetNgcc_HMIAirCdnrAutDrySetNgcc_write) -> HMIAirCdnrAutDrySetNgcc_HMIAirCdnrAutDrySetNgcc/1
  SdbRxSigProc_Runnable_sys/54(HmiAirDistbnRow1LeNgcc_HmiAirDistbnRow1LeNgcc_write) -> HmiAirDistbnRow1LeNgcc_HmiAirDistbnRow1LeNgcc/1
  SdbRxSigProc_Runnable_sys/55(HmiAirDistbnRow1RiNgcc_HmiAirDistbnRow1RiNgcc_write) -> HmiAirDistbnRow1RiNgcc_HmiAirDistbnRow1RiNgcc/1
  SdbRxSigProc_Runnable_sys/56(HmiAutoRow1LeNgcc_HmiAutoRow1LeNgcc_write) -> HmiAutoRow1LeNgcc_HmiAutoRow1LeNgcc/1
  SdbRxSigProc_Runnable_sys/57(HmiAutoRow1RiNgcc_HmiAutoRow1RiNgcc_write) -> HmiAutoRow1RiNgcc_HmiAutoRow1RiNgcc/1
  SdbRxSigProc_Runnable_sys/58(HmiAutOxySetNgcc_HmiAutOxySetNgcc_write) -> HmiAutOxySetNgcc_HmiAutOxySetNgcc/1
  SdbRxSigProc_Runnable_sys/59(HmiCarLockACRecircSetNgcc_HmiCarLockACRecircSetNgcc_write) -> HmiCarLockACRecircSetNgcc_HmiCarLockACRecircSetNgcc/1
  SdbRxSigProc_Runnable_sys/60(HmiClimaRow1LePwrNgcc_HmiClimaRow1LePwrNgcc_write) -> HmiClimaRow1LePwrNgcc_HmiClimaRow1LePwrNgcc/1
  SdbRxSigProc_Runnable_sys/61(HmiClimaRow1PwrNgcc_HmiClimaRow1PwrNgcc_write) -> HmiClimaRow1PwrNgcc_HmiClimaRow1PwrNgcc/1
  SdbRxSigProc_Runnable_sys/62(HmiClimaRow1RiPwrNgcc_HmiClimaRow1RiPwrNgcc_write) -> HmiClimaRow1RiPwrNgcc_HmiClimaRow1RiPwrNgcc/1
  SdbRxSigProc_Runnable_sys/63(HmiClimaRow2PwrNgcc_HmiClimaRow2PwrNgcc_write) -> HmiClimaRow2PwrNgcc_HmiClimaRow2PwrNgcc/1